simulink model "ofdm_txrx_alamouti"  (Simulink 6.3)
settings: Solver=ode45  StartTime=0.0  StopTime=45000  RelTol=1e-3
InitFcn:
  | ofdm_rx_alamouti_init
StartFcn:
  | ofdm_rx_alamouti_init
values from neighbouring files (symbols this model uses):
  CPLength = 16    [ofdm_tx_alamouti_init.m]
  CRCPolynomial = hex2dec('04c11db7')    [calcTxCRC.m]    (also in ofdm_tx_alamouti_init.m)
  hi = zeros(1,length(cci))    [ofdm_rx_alamouti_init.m]
  hr = zeros(1,length(ccr))    [ofdm_rx_alamouti_init.m]
  max_CP_length = 16    [ofdm_tx_alamouti_init.m]
  max_num_baseRateSymbols = 31    [ofdm_tx_alamouti_init.m]
  max_num_subcarriers = 64    [ofdm_tx_alamouti_init.m]
  max_num_trainingSymbols = 15    [ofdm_tx_alamouti_init.m]
  max_OFDM_symbols = 2047    [ofdm_tx_alamouti_init.m]
  modConstellation_qam16 = 0.75*[1 3 -1 -3]./3    [ofdm_tx_alamouti_init.m]
  modConstellation_qam256 = 0.9375*[3 1 5 7 11 9 13 15 -3 -1 -5 -7 -11 -9 -13 -15]./15    [ofdm_tx_alamouti_init.m]
  modConstellation_qam64 = 0.875*[3 1 5 7 -3 -1 -5 -7]./7    [ofdm_tx_alamouti_init.m]
  modConstellation_qpsk = [1 -1]./sqrt(2)    [ofdm_tx_alamouti_init.m]
  numBaseRateSymbols = 2    [ofdm_tx_alamouti_init.m]
  numFullRateSymbols = numFullRateSymbols + mod(numFullRateSymbols, 2)    [ofdm_tx_alamouti_init.m]
  numSubcarriers = 64    [ofdm_tx_alamouti_init.m]
  numTrainingSymbols = 4    [ofdm_tx_alamouti_init.m]
  pkt_crossCorr_thresh = 0.7    [ofdm_rx_alamouti_init.m]
  pkt_energy_thresh = 0    [ofdm_rx_alamouti_init.m]
  pkt_numPayloadBytes = 24+4+20+20+500    (= 568)    [ofdm_tx_alamouti_init.m]
  pktTiming_count_payloadStart = pktTiming_count_lsStart + pktTiming_ls_num*pktTiming_ls_length    [ofdm_rx_init_packetTimingControl.m]
  preamble = 6*[0 shortsyms_10 longsyms_2]    [ofdm_tx_alamouti_init.m]
  preamble_I = real(preamble)    [ofdm_tx_alamouti_init.m]
  preamble_Q = imag(preamble)    [ofdm_tx_alamouti_init.m]
  RAM_init_size = 4096    [ofdm_tx_alamouti_init.m]
  rx_longCorrCountLoad = 251-16    (= 235)    [ofdm_rx_alamouti_init.m]
  rx_longCorrThresh = 3000    [ofdm_rx_alamouti_init.m]
  RxDataScrambling_Seq = [40 198 78 63 82 173 102 245 48 111 172 115 147 230 216 93 72 65 62 2 205 242 122 90 128 83 105 97 73 10 5 252]    [ofdm_tx_alamouti_init.m]
  simOnly_numSamples = length(preamble)+( (numSubcarriers+CPLength)*(numTrainingSymbols + numBaseRateSymbols + numFullRateSymbols) )    [ofdm_tx_alamouti_init.m]
  train = [train train]    [ofdm_tx_alamouti_init.m]
  TxDataScrambling_Seq = [40 198 78 63 82 173 102 245 48 111 172 115 147 230 216 93 72 65 62 2 205 242 122 90 128 83 105 97 73 10 5 252]    [ofdm_tx_alamouti_init.m]
undefined symbols (not defined in the file or in its folder): CRC_table_gen, gcbh, interpFilter_4, xlBlockLoadCallback
libraries: xbsIndex_r4 x4373, dspmlti4 x4, dspsigops x4, commchan3 x2, simulink x2, commrflib2 x2, commsink2 x2, dspsrcs4 x1
(part 13 of 39)

== system ofdm_txrx_alamouti/OFDM Rx Alamouti/Equalizer & Packetizer/Phase Adjust/ComplexMult_x1 ==
  Re[A] : Inport
  Im[A] : Inport  Port=2
  Re[B] : Inport  Port=3
  Im[B] : Inport  Port=4
  Add1 : xbsIndex_r4/AddSub  mode=Addition  use_carryin=off  use_carryout=off  en=off  latency=0  precision=User Defined  arith_type="Signed  (2's comp)"  n_bits=18  bin_pt=15  quantization=Truncate  overflow=Flag as error  dbl_ovrd=off  use_behavioral_HDL=on  pipelined=off  use_rpm=on  block_type=addsub
      settings: xl_area=[9 0 0 16 0 0 0]  block_version=VER_STRING_GOES_HERE
  Add2 : xbsIndex_r4/AddSub  mode=Addition  use_carryin=off  use_carryout=off  en=off  latency=0  precision=User Defined  arith_type="Signed  (2's comp)"  n_bits=18  bin_pt=15  quantization=Truncate  overflow=Flag as error  dbl_ovrd=off  use_behavioral_HDL=on  pipelined=off  use_rpm=on  block_type=addsub
      settings: xl_area=[9 0 0 16 0 0 0]  block_version=VER_STRING_GOES_HERE
  Counter1 : xbsIndex_r4/Counter  Orientation=down  cnt_type=Free Running  cnt_to=4  operation=Up  start_count=0  cnt_by_val=1  arith_type=Unsigned  n_bits=1  bin_pt=0  load_pin=off  rst=off  en=off  explicit_period=on  period=1  dbl_ovrd=off  use_behavioral_HDL=off  use_rpm=on  block_type=counter
      settings: xl_area=[3 3 0 4 0 0 0]  block_version=VER_STRING_GOES_HERE
  Delay3 : xbsIndex_r4/Delay  en=off  latency=1  dbl_ovrd=off  reg_retiming=off  block_type=delay
      settings: infoedit=Hardware notes: A delay line is a chain, each link of which is an SRL16 followed by a flip-flop. If register retiming is enabled, the delay line is a chain of flip-flops.  xl_area=[8 16 0 0 0 0 0]  block_version=VER_STRING_GOES_HERE
  Delay5 : xbsIndex_r4/Delay  en=off  latency=1  dbl_ovrd=off  reg_retiming=off  block_type=delay
      settings: infoedit=Hardware notes: A delay line is a chain, each link of which is an SRL16 followed by a flip-flop. If register retiming is enabled, the delay line is a chain of flip-flops.  xl_area=[8 16 0 0 0 0 0]  block_version=VER_STRING_GOES_HERE
  Down Sample1 : xbsIndex_r4/Down Sample  sample_ratio=2  sample_phase=First Value of Frame  en=off  latency=1  dbl_ovrd=off  block_type=dsamp
      settings: xl_area=[9 17 0 0 0 0 0]  block_version=VER_STRING_GOES_HERE
  Down Sample3 : xbsIndex_r4/Down Sample  sample_ratio=2  sample_phase=First Value of Frame  en=off  latency=1  dbl_ovrd=off  block_type=dsamp
      settings: xl_area=[9 17 0 0 0 0 0]  block_version=VER_STRING_GOES_HERE
  Mult1 : xbsIndex_r4/Mult  precision=User Defined  arith_type="Signed  (2's comp)"  n_bits=18  bin_pt=16  quantization=Truncate  overflow=Flag as error  en=off  latency=1  dbl_ovrd=off  use_behavioral_HDL=off  use_embedded=on  opt=Speed  optimum_pipeline=off  use_rpm=on  placement_style=Rectangular shape  pipeline=off  block_type=mult
      settings: infoedit=Hardware notes: To use the internal pipeline stage of the dedicated multiplier you must select 'Pipeline for maximum performance'.  xl_area=[14 27 0 27 0 1 0]  block_version=VER_STRING_GOES_HERE
  Mult2 : xbsIndex_r4/Mult  precision=User Defined  arith_type="Signed  (2's comp)"  n_bits=18  bin_pt=16  quantization=Truncate  overflow=Flag as error  en=off  latency=1  dbl_ovrd=off  use_behavioral_HDL=off  use_embedded=on  opt=Speed  optimum_pipeline=off  use_rpm=on  placement_style=Rectangular shape  pipeline=off  block_type=mult
      settings: infoedit=Hardware notes: To use the internal pipeline stage of the dedicated multiplier you must select 'Pipeline for maximum performance'.  xl_area=[14 27 0 27 0 1 0]  block_version=VER_STRING_GOES_HERE
  Mux{2..5} (4 alike) : xbsIndex_r4/Mux  inputs=2  en=off  latency=0  precision=Full  arith_type="Signed  (2's comp)"  n_bits=16  bin_pt=13  quantization=Truncate  overflow=Wrap  dbl_ovrd=off  block_type=mux
      settings: xl_area=[14 0 0 28 0 0 0]  block_version=VER_STRING_GOES_HERE
  Negate2 : xbsIndex_r4/Negate  precision=Full  arith_type="Signed  (2's comp)"  n_bits=14  bin_pt=13  quantization=Truncate  overflow=Flag as error  en=off  latency=0  dbl_ovrd=off  block_type=negate
      settings: xl_area=[7 0 0 14 0 0 0]  block_version=VER_STRING_GOES_HERE
  Up Sample{5..8} (4 alike) : xbsIndex_r4/Up Sample  sample_ratio=2  copy_samples=on  dbl_ovrd=off  block_type=usamp
      settings: infoedit="Up samples input data.  Inserted values  can be zeros or copies of the most recent input sample.<P><P>Hardware notes: No hardware is needed if inserted values are copies of the input sample; otherwise, a mux and single bit flip-flop are used."  xl_area=[0 0 0 0 0 0 0]  block_version=VER_STRING_GOES_HERE
  Re : Outport
  Im : Outport  Port=2
  wires:
  Down Sample1/1 -> Im/1
  Down Sample3/1 -> Re/1
  Add2/1 -> Down Sample3/1
  Add1/1 -> Down Sample1/1
  Delay5/1 -> Add1/2
  Mult2/1 -> Add1/1, Delay5/1
  Negate2/1 -> Mux3/3
  Im[B]/1 -> Up Sample6/1
  Re[B]/1 -> Up Sample8/1
  Im[A]/1 -> Up Sample5/1
  Re[A]/1 -> Up Sample7/1
  Up Sample8/1 -> Mux4/3, Mux2/2
  Up Sample7/1 -> Mux5/2, Mux3/2
  Mux4/1 -> Mult2/2
  Mux5/1 -> Mult2/1
  Mux2/1 -> Mult1/2
  Mux3/1 -> Mult1/1
  Up Sample6/1 -> Mux4/2, Mux2/3
  Up Sample5/1 -> Mux5/3, Negate2/1
  Mult1/1 -> Delay3/1, Add2/1
  Delay3/1 -> Add2/2
  Counter1/1 -> Mux4/1, Mux5/1, Mux2/1, Mux3/1

== system ofdm_txrx_alamouti/OFDM Rx Alamouti/Equalizer & Packetizer/Phase Adjust/ComplexMult_x2 ==
  Re[A] : Inport
  Im[A] : Inport  Port=2
  Re[B] : Inport  Port=3
  Im[B] : Inport  Port=4
  Add1 : xbsIndex_r4/AddSub  mode=Addition  use_carryin=off  use_carryout=off  en=off  latency=0  precision=User Defined  arith_type="Signed  (2's comp)"  n_bits=18  bin_pt=15  quantization=Truncate  overflow=Flag as error  dbl_ovrd=off  use_behavioral_HDL=on  pipelined=off  use_rpm=on  block_type=addsub
      settings: xl_area=[9 0 0 16 0 0 0]  block_version=VER_STRING_GOES_HERE
  Add2 : xbsIndex_r4/AddSub  mode=Addition  use_carryin=off  use_carryout=off  en=off  latency=0  precision=User Defined  arith_type="Signed  (2's comp)"  n_bits=18  bin_pt=15  quantization=Truncate  overflow=Flag as error  dbl_ovrd=off  use_behavioral_HDL=on  pipelined=off  use_rpm=on  block_type=addsub
      settings: xl_area=[9 0 0 16 0 0 0]  block_version=VER_STRING_GOES_HERE
  Counter1 : xbsIndex_r4/Counter  Orientation=down  cnt_type=Free Running  cnt_to=4  operation=Up  start_count=0  cnt_by_val=1  arith_type=Unsigned  n_bits=1  bin_pt=0  load_pin=off  rst=off  en=off  explicit_period=on  period=1  dbl_ovrd=off  use_behavioral_HDL=off  use_rpm=on  block_type=counter
      settings: xl_area=[3 3 0 4 0 0 0]  block_version=VER_STRING_GOES_HERE
  Delay3 : xbsIndex_r4/Delay  en=off  latency=1  dbl_ovrd=off  reg_retiming=off  block_type=delay
      settings: infoedit=Hardware notes: A delay line is a chain, each link of which is an SRL16 followed by a flip-flop. If register retiming is enabled, the delay line is a chain of flip-flops.  xl_area=[8 16 0 0 0 0 0]  block_version=VER_STRING_GOES_HERE
  Delay5 : xbsIndex_r4/Delay  en=off  latency=1  dbl_ovrd=off  reg_retiming=off  block_type=delay
      settings: infoedit=Hardware notes: A delay line is a chain, each link of which is an SRL16 followed by a flip-flop. If register retiming is enabled, the delay line is a chain of flip-flops.  xl_area=[8 16 0 0 0 0 0]  block_version=VER_STRING_GOES_HERE
  Down Sample1 : xbsIndex_r4/Down Sample  sample_ratio=2  sample_phase=First Value of Frame  en=off  latency=1  dbl_ovrd=off  block_type=dsamp
      settings: xl_area=[9 17 0 0 0 0 0]  block_version=VER_STRING_GOES_HERE
  Down Sample3 : xbsIndex_r4/Down Sample  sample_ratio=2  sample_phase=First Value of Frame  en=off  latency=1  dbl_ovrd=off  block_type=dsamp
      settings: xl_area=[9 17 0 0 0 0 0]  block_version=VER_STRING_GOES_HERE
  Mult1 : xbsIndex_r4/Mult  precision=User Defined  arith_type="Signed  (2's comp)"  n_bits=18  bin_pt=16  quantization=Truncate  overflow=Flag as error  en=off  latency=1  dbl_ovrd=off  use_behavioral_HDL=off  use_embedded=on  opt=Speed  optimum_pipeline=off  use_rpm=on  placement_style=Rectangular shape  pipeline=off  block_type=mult
      settings: infoedit=Hardware notes: To use the internal pipeline stage of the dedicated multiplier you must select 'Pipeline for maximum performance'.  xl_area=[14 27 0 27 0 1 0]  block_version=VER_STRING_GOES_HERE
  Mult2 : xbsIndex_r4/Mult  precision=User Defined  arith_type="Signed  (2's comp)"  n_bits=18  bin_pt=16  quantization=Truncate  overflow=Flag as error  en=off  latency=1  dbl_ovrd=off  use_behavioral_HDL=off  use_embedded=on  opt=Speed  optimum_pipeline=off  use_rpm=on  placement_style=Rectangular shape  pipeline=off  block_type=mult
      settings: infoedit=Hardware notes: To use the internal pipeline stage of the dedicated multiplier you must select 'Pipeline for maximum performance'.  xl_area=[14 27 0 27 0 1 0]  block_version=VER_STRING_GOES_HERE
  Mux{2..5} (4 alike) : xbsIndex_r4/Mux  inputs=2  en=off  latency=0  precision=Full  arith_type="Signed  (2's comp)"  n_bits=16  bin_pt=13  quantization=Truncate  overflow=Wrap  dbl_ovrd=off  block_type=mux
      settings: xl_area=[14 0 0 28 0 0 0]  block_version=VER_STRING_GOES_HERE
  Negate2 : xbsIndex_r4/Negate  precision=Full  arith_type="Signed  (2's comp)"  n_bits=14  bin_pt=13  quantization=Truncate  overflow=Flag as error  en=off  latency=0  dbl_ovrd=off  block_type=negate
      settings: xl_area=[7 0 0 14 0 0 0]  block_version=VER_STRING_GOES_HERE
  Up Sample{5..8} (4 alike) : xbsIndex_r4/Up Sample  sample_ratio=2  copy_samples=on  dbl_ovrd=off  block_type=usamp
      settings: infoedit="Up samples input data.  Inserted values  can be zeros or copies of the most recent input sample.<P><P>Hardware notes: No hardware is needed if inserted values are copies of the input sample; otherwise, a mux and single bit flip-flop are used."  xl_area=[0 0 0 0 0 0 0]  block_version=VER_STRING_GOES_HERE
  Re : Outport
  Im : Outport  Port=2
  wires:
  Counter1/1 -> Mux3/1, Mux2/1, Mux5/1, Mux4/1
  Delay3/1 -> Add2/2
  Mult1/1 -> Add2/1, Delay3/1
  Up Sample5/1 -> Negate2/1, Mux5/3
  Up Sample6/1 -> Mux2/3, Mux4/2
  Mux3/1 -> Mult1/1
  Mux2/1 -> Mult1/2
  Mux5/1 -> Mult2/1
  Mux4/1 -> Mult2/2
  Up Sample7/1 -> Mux3/2, Mux5/2
  Up Sample8/1 -> Mux2/2, Mux4/3
  Re[A]/1 -> Up Sample7/1
  Im[A]/1 -> Up Sample5/1
  Re[B]/1 -> Up Sample8/1
  Im[B]/1 -> Up Sample6/1
  Negate2/1 -> Mux3/3
  Mult2/1 -> Delay5/1, Add1/1
  Delay5/1 -> Add1/2
  Add1/1 -> Down Sample1/1
  Add2/1 -> Down Sample3/1
  Down Sample3/1 -> Re/1
  Down Sample1/1 -> Im/1

== system ofdm_txrx_alamouti/OFDM Rx Alamouti/Equalizer & Packetizer/Phase Adjust/Phase Calc ==
  Phase : Inport
  Convert4 : xbsIndex_r4/Convert  arith_type="Signed  (2's comp)"  n_bits=15  bin_pt=10  quantization=Truncate  overflow=Flag as error  latency=0  dbl_ovrd=off  pipeline=off  block_type=convert
      settings: xl_area=[0, 0, 0, 0, 0, 0, 0]  block_version=VER_STRING_GOES_HERE
  Down Sample1 : xbsIndex_r4/Down Sample  sample_ratio=2  sample_phase="Last Value of Frame  (most efficient)"  en=off  latency=1  dbl_ovrd=off  block_type=dsamp
      settings: block_version=VER_STRING_GOES_HERE
  Mult1 : xbsIndex_r4/Mult  precision=User Defined  arith_type="Signed  (2's comp)"  n_bits=12  bin_pt=10  quantization=Truncate  overflow=Flag as error  en=off  latency=1  dbl_ovrd=off  use_behavioral_HDL=off  use_embedded=on  opt=Speed  optimum_pipeline=off  use_rpm=off  placement_style=Rectangular shape  pipeline=on  block_type=mult
      settings: infoedit=Hardware notes: To use the internal pipeline stage of the dedicated multiplier you must select 'Pipeline for maximum performance'.  block_version=VER_STRING_GOES_HERE
  Rx_PhaseNoiseTrack_K : xbsIndex_r4/From Register  shared_memory_name='Rx_PhaseNoiseTrack_K'  init=Rx_PhaseNoiseTrack_K  period=1  ownership=Locally owned and initialized  arith_type=Unsigned  n_bits=32  bin_pt=26  dbl_ovrd=off  block_type=fromreg
      settings: AttributesFormatString=<< %<shared_memory_name> >>  infoedit="Register block that reads data to a shared memory register.  Delay of one sample period."  block_version=VER_STRING_GOES_HERE
  " Phase" : Outport
  wires:
  Phase/1 -> Convert4/1
  Down Sample1/1 -> Mult1/2
  Rx_PhaseNoiseTrack_K/1 -> Down Sample1/1
  Mult1/1 -> " Phase"/1
  Convert4/1 -> Mult1/1

== system ofdm_txrx_alamouti/OFDM Rx Alamouti/Equalizer & Packetizer/Phase Noise Tracker/Coarse Tracking ==
  Phase Err : Inport
  Rst : Inport  Port=2
  Vin : Inport  Port=3
  Accumulator1 : xbsIndex_r4/Accumulator  operation=Add  n_bits=32  overflow=Wrap  scale=1  rst=on  hasbypass=off  en=on  dbl_ovrd=off  use_behavioral_HDL=on  block_type=accum
      settings: infoedit="Adder or subtractor-based accumulator.   Output type and binary point position match the input.<P><P>Hardware notes: When \"Reinitialize with input 'b' on reset\" is selected, the accumulator is forced to run at the system rate even if the input 'b' is running at a slower rate."  block_version=VER_STRING_GOES_HERE
  AddSub1 : xbsIndex_r4/AddSub  mode=Subtraction  use_carryin=off  use_carryout=off  en=off  latency=0  precision=User Defined  arith_type="Signed  (2's comp)"  n_bits=32  bin_pt=28  quantization=Truncate  overflow=Wrap  dbl_ovrd=off  use_behavioral_HDL=off  pipelined=off  use_rpm=on  block_type=addsub
      settings: block_version=VER_STRING_GOES_HERE
  Coarse Phase Tracker : Scope  NumInputPorts=6
      settings: YMin=-1~-1~-1~-1~-1~-1  YMax=1~1~1~1~1~1  SaveName=ScopeData40
  Delay : xbsIndex_r4/Delay  en=off  latency=3  dbl_ovrd=off  reg_retiming=off  block_type=delay
      settings: infoedit=Hardware notes: A delay line is a chain, each link of which is an SRL16 followed by a flip-flop. If register retiming is enabled, the delay line is a chain of flip-flops.  block_version=VER_STRING_GOES_HERE
  Delay1 : xbsIndex_r4/Delay  en=off  latency=1  dbl_ovrd=off  reg_retiming=off  block_type=delay
      settings: infoedit=Hardware notes: A delay line is a chain, each link of which is an SRL16 followed by a flip-flop. If register retiming is enabled, the delay line is a chain of flip-flops.  block_version=VER_STRING_GOES_HERE
  Display : Display
  Down Sample2 : xbsIndex_r4/Down Sample  sample_ratio=2  sample_phase="Last Value of Frame  (most efficient)"  en=off  latency=1  dbl_ovrd=off  block_type=dsamp
      settings: block_version=VER_STRING_GOES_HERE
  Gateway Out{1..6} (6 alike) : xbsIndex_r4/Gateway Out  hdl_port=off  timing_constraint=None  locs_specified=off  LOCs={}  block_type=gatewayout
      settings: infoedit="Gateway out block.  Converts Xilinx fixed point inputs into ouputs of type Simulink integer, double, or fixed point.<P><P>Hardware notes:  In hardware these blocks become top level output ports or are discarded, depending on how they are configured."  block_version=VER_STRING_GOES_HERE
  Mult2 : xbsIndex_r4/Mult  precision=User Defined  arith_type="Signed  (2's comp)"  n_bits=32  bin_pt=28  quantization=Truncate  overflow=Wrap  en=off  latency=3  dbl_ovrd=off  use_behavioral_HDL=off  use_embedded=on  opt=Speed  optimum_pipeline=off  use_rpm=off  placement_style=Rectangular shape  pipeline=on  block_type=mult
      settings: infoedit=Hardware notes: To use the internal pipeline stage of the dedicated multiplier you must select 'Pipeline for maximum performance'.  block_version=VER_STRING_GOES_HERE
  Register1 : xbsIndex_r4/Register  Orientation=left  init=0  rst=on  en=on  dbl_ovrd=off  block_type=register
      settings: block_version=8.2.02
  Register2 : xbsIndex_r4/Register  init=0  rst=on  en=on  dbl_ovrd=off  block_type=register
      settings: block_version=8.2.02
  Rx_PhaseNoiseTrack_Kalpha : xbsIndex_r4/From Register  shared_memory_name='Rx_PhaseNoiseTrack_Kalpha'  init=Rx_PhaseNoiseTrack_Kalpha  period=1  ownership=Locally owned and initialized  arith_type=Unsigned  n_bits=32  bin_pt=32  dbl_ovrd=off  block_type=fromreg
      settings: AttributesFormatString=<< %<shared_memory_name> >>  infoedit="Register block that reads data to a shared memory register.  Delay of one sample period."  block_version=VER_STRING_GOES_HERE
  phi : Outport
  theta_coarse : Outport  Port=2
  vout : Outport  Port=3
  wires:
  Gateway Out6/1 -> Coarse Phase Tracker/6
  Gateway Out5/1 -> Coarse Phase Tracker/5
  Gateway Out4/1 -> Coarse Phase Tracker/4
  Gateway Out3/1 -> Coarse Phase Tracker/3
  Gateway Out1/1 -> Coarse Phase Tracker/2
  Gateway Out2/1 -> Coarse Phase Tracker/1
  Phase Err/1 -> AddSub1/1, Gateway Out2/1
  Rx_PhaseNoiseTrack_Kalpha/1 -> Down Sample2/1, Display/1
  Down Sample2/1 -> Mult2/2
  Mult2/1 -> Accumulator1/1
  Delay/1 -> Accumulator1/3
  Accumulator1/1 -> theta_coarse/1, Register1/1, Gateway Out3/1
  AddSub1/1 -> Register2/1
  Register2/1 -> phi/1, Mult2/1, Gateway Out1/1
  Rst/1 -> Register2/2, Register1/2, Accumulator1/2, Gateway Out6/1
  Vin/1 -> Register2/3, Register1/3, Delay/1, Delay1/1, Gateway Out5/1
  Register1/1 -> AddSub1/2, Gateway Out4/1
  Delay1/1 -> vout/1

== system ofdm_txrx_alamouti/OFDM Rx Alamouti/Equalizer & Packetizer/Phase Noise Tracker/Fine Tracking ==
  note: Overflow potential before/after accumulator?
  phi : Inport
  theta_coarse : Inport  Port=2
  Vin : Inport  Port=3
  Rst : Inport  Port=4
  Sym Index : Inport  Port=5
  Accumulator1 : xbsIndex_r4/Accumulator  operation=Add  n_bits=32  overflow=Flag as error  scale=1  rst=on  hasbypass=off  en=on  dbl_ovrd=off  use_behavioral_HDL=on  block_type=accum
      settings: infoedit="Adder or subtractor-based accumulator.   Output type and binary point position match the input.<P><P>Hardware notes: When \"Reinitialize with input 'b' on reset\" is selected, the accumulator is forced to run at the system rate even if the input 'b' is running at a slower rate."  block_version=VER_STRING_GOES_HERE
  AddSub1 : xbsIndex_r4/AddSub  mode=Addition  use_carryin=off  use_carryout=off  en=off  latency=0  precision=User Defined  arith_type="Signed  (2's comp)"  n_bits=32  bin_pt=28  quantization=Truncate  overflow=Wrap  dbl_ovrd=off  use_behavioral_HDL=off  pipelined=off  use_rpm=on  block_type=addsub
      settings: block_version=VER_STRING_GOES_HERE
  AddSub2 : xbsIndex_r4/AddSub  mode=Addition  use_carryin=off  use_carryout=off  en=off  latency=0  precision=User Defined  arith_type="Signed  (2's comp)"  n_bits=32  bin_pt=28  quantization=Truncate  overflow=Wrap  dbl_ovrd=off  use_behavioral_HDL=off  pipelined=off  use_rpm=on  block_type=addsub
      settings: block_version=VER_STRING_GOES_HERE
  Constant7 : xbsIndex_r4/Constant  arith_type=Unsigned  const=0.5  n_bits=4  bin_pt=2  explicit_period=on  period=1  equ=P=C  opselect=C  inp2=PCIN>>17  opr=+  inp1=P  carry=CIN  dbl_ovrd=off  block_type=constant
      settings: block_version=VER_STRING_GOES_HERE
  Delay : xbsIndex_r4/Delay  en=off  latency=3  dbl_ovrd=off  reg_retiming=off  block_type=delay
      settings: infoedit=Hardware notes: A delay line is a chain, each link of which is an SRL16 followed by a flip-flop. If register retiming is enabled, the delay line is a chain of flip-flops.  block_version=VER_STRING_GOES_HERE
  Delay1 : xbsIndex_r4/Delay  en=off  latency=4  dbl_ovrd=off  reg_retiming=off  block_type=delay
      settings: infoedit=Hardware notes: A delay line is a chain, each link of which is an SRL16 followed by a flip-flop. If register retiming is enabled, the delay line is a chain of flip-flops.  block_version=VER_STRING_GOES_HERE
  Display : Display
  Display1 : Display
  Down Sample1 : xbsIndex_r4/Down Sample  sample_ratio=2  sample_phase="Last Value of Frame  (most efficient)"  en=off  latency=1  dbl_ovrd=off  block_type=dsamp
      settings: block_version=VER_STRING_GOES_HERE
  Down Sample2 : xbsIndex_r4/Down Sample  sample_ratio=2  sample_phase="Last Value of Frame  (most efficient)"  en=off  latency=1  dbl_ovrd=off  block_type=dsamp
      settings: block_version=VER_STRING_GOES_HERE
  Fine Phase Tracker : Scope  NumInputPorts=6
      settings: YMin=-1~-1~-1~-1~-1~-1  YMax=1~1~1~1~1~1  SaveName=ScopeData40
  Gateway Out{1..6} (6 alike) : xbsIndex_r4/Gateway Out  hdl_port=off  timing_constraint=None  locs_specified=off  LOCs={}  block_type=gatewayout
      settings: infoedit="Gateway out block.  Converts Xilinx fixed point inputs into ouputs of type Simulink integer, double, or fixed point.<P><P>Hardware notes:  In hardware these blocks become top level output ports or are discarded, depending on how they are configured."  block_version=VER_STRING_GOES_HERE
  Mult1 : xbsIndex_r4/Mult  precision=User Defined  arith_type="Signed  (2's comp)"  n_bits=32  bin_pt=28  quantization=Truncate  overflow=Wrap  en=off  latency=3  dbl_ovrd=off  use_behavioral_HDL=off  use_embedded=on  opt=Speed  optimum_pipeline=off  use_rpm=off  placement_style=Rectangular shape  pipeline=on  block_type=mult
      settings: infoedit=Hardware notes: To use the internal pipeline stage of the dedicated multiplier you must select 'Pipeline for maximum performance'.  block_version=VER_STRING_GOES_HERE
  Mult2 : xbsIndex_r4/Mult  precision=User Defined  arith_type="Signed  (2's comp)"  n_bits=32  bin_pt=24  quantization=Truncate  overflow=Wrap  en=off  latency=3  dbl_ovrd=off  use_behavioral_HDL=off  use_embedded=on  opt=Speed  optimum_pipeline=off  use_rpm=off  placement_style=Rectangular shape  pipeline=on  block_type=mult
      settings: infoedit=Hardware notes: To use the internal pipeline stage of the dedicated multiplier you must select 'Pipeline for maximum performance'.  block_version=VER_STRING_GOES_HERE
  Mult3 : xbsIndex_r4/Mult  precision=User Defined  arith_type="Signed  (2's comp)"  n_bits=32  bin_pt=28  quantization=Truncate  overflow=Wrap  en=off  latency=3  dbl_ovrd=off  use_behavioral_HDL=off  use_embedded=on  opt=Speed  optimum_pipeline=off  use_rpm=off  placement_style=Rectangular shape  pipeline=on  block_type=mult
      settings: infoedit=Hardware notes: To use the internal pipeline stage of the dedicated multiplier you must select 'Pipeline for maximum performance'.  block_version=VER_STRING_GOES_HERE
  ROM : xbsIndex_r4/ROM  depth=floor(max_OFDM_symbols/2)  initVector=1./[1:floor(max_OFDM_symbols/2)]  distributed_mem=Distributed memory  rst=off  init_reg=0  en=off  latency=1  arith_type=Unsigned  n_bits=8  bin_pt=8  dbl_ovrd=off  optimize=Area  use_rpm=on  block_type=sprom
      settings: block_version=8.2.02
  Register2 : xbsIndex_r4/Register  init=0  rst=on  en=on  dbl_ovrd=off  block_type=register
      settings: block_version=8.2.02
  Slice9 : xbsIndex_r4/Slice  nbits=ceil(log2(max_OFDM_symbols))-1  boolean_output=off  mode=Upper Bit Location + Width  bit1=0  base1=MSB of Input  bit0=0  base0=LSB of Input  dbl_ovrd=off  block_type=slice
      settings: infoedit="Extracts a given range of bits from each input sample and presents it at the output.  The output type is ordinarily unsigned with binary point at zero, but can be Boolean when the slice is one bit wide.<P><P>Hardware notes: In hardware this block costs nothing."  block_version=VER_STRING_GOES_HERE
  theta : Outport
  wires:
  AddSub1/1 -> Register2/1
  Delay/1 -> Accumulator1/3, Delay1/1
  Mult2/1 -> Accumulator1/1
  Down Sample2/1 -> Mult2/2
  theta_coarse/1 -> AddSub1/1, Gateway Out1/1
  Gateway Out2/1 -> Fine Phase Tracker/1
  Gateway Out1/1 -> Fine Phase Tracker/2
  Gateway Out3/1 -> Fine Phase Tracker/3
  Gateway Out4/1 -> Fine Phase Tracker/4
  Gateway Out5/1 -> Fine Phase Tracker/5
  Gateway Out6/1 -> Fine Phase Tracker/6
  phi/1 -> Mult2/1, Mult1/2, Gateway Out2/1
  Down Sample1/1 -> Mult1/1
  Constant7/1 -> Down Sample1/1, Display1/1, Display/1, Down Sample2/1
  Mult1/1 -> AddSub2/1
  Accumulator1/1 -> Mult3/1
  ROM/1 -> Mult3/2, Gateway Out5/1
  Sym Index/1 -> Slice9/1
  Mult3/1 -> AddSub2/2, Gateway Out6/1
  AddSub2/1 -> AddSub1/2, Gateway Out3/1
  Rst/1 -> Accumulator1/2, Register2/2
  Vin/1 -> Delay/1
  Delay1/1 -> Register2/3
  Register2/1 -> Gateway Out4/1, theta/1
  Slice9/1 -> ROM/1

== system ofdm_txrx_alamouti/OFDM Rx Alamouti/Equalizer & Packetizer/Pilot_Extractor/Phase Calc & Combining ==
  Rst : Inport
  I : Inport  Port=2
  Q : Inport  Port=3
  mag(H) : Inport  Port=4
  Vin : Inport  Port=5
  1/sum(mag(H)) : Subsystem
  Accumulator : xbsIndex_r4/Accumulator  operation=Add  n_bits=24  overflow=Flag as error  scale=1  rst=on  hasbypass=off  en=on  dbl_ovrd=off  use_behavioral_HDL=on  block_type=accum
      settings: infoedit="Adder or subtractor-based accumulator.   Output type and binary point position match the input.<P><P>Hardware notes: When \"Reinitialize with input 'b' on reset\" is selected, the accumulator is forced to run at the system rate even if the input 'b' is running at a slower rate."  block_version=8.2.02
  Constant : xbsIndex_r4/Constant  arith_type=Unsigned  const=0  n_bits=1  bin_pt=0  explicit_period=off  period=1  equ=P=C  opselect=C  inp2=PCIN>>17  opr=+  inp1=P  carry=CIN  dbl_ovrd=off  block_type=constant
      settings: block_version=8.2.02
  Counter : xbsIndex_r4/Counter  cnt_type=Free Running  cnt_to=Inf  operation=Up  start_count=0  cnt_by_val=1  arith_type=Unsigned  n_bits=3  bin_pt=0  load_pin=off  rst=on  en=on  explicit_period=off  period=1  dbl_ovrd=off  use_behavioral_HDL=off  use_rpm=off  block_type=counter
      settings: block_version=8.2.02
  Delay1 : xbsIndex_r4/Delay  en=off  latency=8  dbl_ovrd=off  reg_retiming=off  block_type=delay
      settings: infoedit=Hardware notes: A delay line is a chain, each link of which is an SRL16 followed by a flip-flop. If register retiming is enabled, the delay line is a chain of flip-flops.  block_version=8.2.02
  Delay2 : xbsIndex_r4/Delay  en=off  latency=2  dbl_ovrd=off  reg_retiming=off  block_type=delay
      settings: infoedit=Hardware notes: A delay line is a chain, each link of which is an SRL16 followed by a flip-flop. If register retiming is enabled, the delay line is a chain of flip-flops.  block_version=8.2.02
  Delay3 : xbsIndex_r4/Delay  en=off  latency=8  dbl_ovrd=off  reg_retiming=off  block_type=delay
      settings: infoedit=Hardware notes: A delay line is a chain, each link of which is an SRL16 followed by a flip-flop. If register retiming is enabled, the delay line is a chain of flip-flops.  block_version=8.2.02
  Delay5 : xbsIndex_r4/Delay  en=off  latency=3  dbl_ovrd=off  reg_retiming=off  block_type=delay
      settings: infoedit=Hardware notes: A delay line is a chain, each link of which is an SRL16 followed by a flip-flop. If register retiming is enabled, the delay line is a chain of flip-flops.  block_version=8.2.02
  Gateway Out{1..3,7..11} (8 alike) : xbsIndex_r4/Gateway Out  hdl_port=off  timing_constraint=None  locs_specified=off  LOCs={}  block_type=gatewayout
      settings: infoedit="Gateway out block.  Converts Xilinx fixed point inputs into ouputs of type Simulink integer, double, or fixed point.<P><P>Hardware notes:  In hardware these blocks become top level output ports or are discarded, depending on how they are configured."  block_version=VER_STRING_GOES_HERE
  Logical : xbsIndex_r4/Logical  logical_function=AND  inputs=2  en=off  latency=0  precision=Full  arith_type=Unsigned  n_bits=16  bin_pt=0  align_bp=on  dbl_ovrd=off  block_type=logical
      settings: block_version=8.2.02
  Mult : xbsIndex_r4/Mult  precision=User Defined  arith_type="Signed  (2's comp)"  n_bits=21  bin_pt=16  quantization=Truncate  overflow=Flag as error  en=off  latency=2  dbl_ovrd=off  use_behavioral_HDL=off  use_embedded=on  opt=Speed  optimum_pipeline=off  use_rpm=on  placement_style=Rectangular shape  pipeline=on  block_type=mult
      settings: infoedit=Hardware notes: To use the internal pipeline stage of the dedicated multiplier you must select 'Pipeline for maximum performance'.  block_version=8.2.02
  Mult1 : xbsIndex_r4/Mult  precision=User Defined  arith_type="Signed  (2's comp)"  n_bits=21  bin_pt=16  quantization=Truncate  overflow=Saturate  en=off  latency=2  dbl_ovrd=off  use_behavioral_HDL=off  use_embedded=on  opt=Speed  optimum_pipeline=off  use_rpm=on  placement_style=Rectangular shape  pipeline=on  block_type=mult
      settings: infoedit=Hardware notes: To use the internal pipeline stage of the dedicated multiplier you must select 'Pipeline for maximum performance'.  block_version=8.2.02
  Phase Calc : Subsystem
  Pilot Phase Combining : Scope  NumInputPorts=8
      settings: TimeRange=5000  YMin=-1~-1~-1~2.29892~-1~-0.0190659~-1~-0.0461426  YMax=1~1~1~2.54092~1~-0.0172501~1~-0.041748  SaveName=ScopeData59
  Register : xbsIndex_r4/Register  init=0  rst=on  en=on  dbl_ovrd=off  block_type=register
      settings: block_version=8.2.02
  Relational3 : xbsIndex_r4/Relational  mode=a=b  en=off  latency=0  dbl_ovrd=off  block_type=relational
      settings: xl_area=[6 0 0 11 0 0 0]  block_version=VER_STRING_GOES_HERE
  PhaseEst : Outport
  Vout : Outport  Port=2
  wires:
  I/1 -> Phase Calc/1(I)
  Q/1 -> Phase Calc/2(Q)
  Phase Calc/1(Phase) -> Mult/1
  mag(H)/1 -> Delay3/1, 1/sum(mag(H))/1(mag(H))
  Mult/1 -> Accumulator/1
  Vin/1 -> Delay1/1, 1/sum(mag(H))/2(Vin), Logical/2, Counter/2, Gateway Out1/1
  Delay1/1 -> Delay2/1
  Delay2/1 -> Accumulator/3
  Accumulator/1 -> Mult1/2, Gateway Out9/1
  Gateway Out11/1 -> Pilot Phase Combining/4
  Gateway Out10/1 -> Pilot Phase Combining/5
  Gateway Out9/1 -> Pilot Phase Combining/6
  Gateway Out8/1 -> Pilot Phase Combining/7
  Gateway Out7/1 -> Pilot Phase Combining/8
  Delay3/1 -> Mult/2
  Counter/1 -> Relational3/2, 1/sum(mag(H))/3(PilotNum), Gateway Out3/1
  Rst/1 -> Counter/1, Gateway Out2/1
  Mult1/1 -> PhaseEst/1, Gateway Out7/1
  Register/1 -> Mult1/1
  1/sum(mag(H))/1(Q) -> Register/1, Gateway Out11/1
  1/sum(mag(H))/2(Vout) -> Register/3, Gateway Out10/1, Delay5/1
  Delay5/1 -> Vout/1, Gateway Out8/1
  Constant/1 -> Relational3/1
  Relational3/1 -> Logical/1
  Logical/1 -> Register/2, Accumulator/2
  Gateway Out2/1 -> Pilot Phase Combining/1
  Gateway Out1/1 -> Pilot Phase Combining/2
  Gateway Out3/1 -> Pilot Phase Combining/3

== system ofdm_txrx_alamouti/OFDM Rx Alamouti/Equalizer & Packetizer/Pilot_Extractor/Pilot Descrambling ==
  Sym Index : Inport
  Rst : Inport  Port=2
  "I " : Inport  Port=3
  "Q " : Inport  Port=4
  FFT Index : Inport  Port=5
  Vin : Inport  Port=6
  Gateway Out{7..14} (8 alike) : xbsIndex_r4/Gateway Out  hdl_port=off  timing_constraint=None  locs_specified=off  LOCs={}  block_type=gatewayout
      settings: infoedit="Gateway out block.  Converts Xilinx fixed point inputs into ouputs of type Simulink integer, double, or fixed point.<P><P>Hardware notes:  In hardware these blocks become top level output ports or are discarded, depending on how they are configured."  block_version=VER_STRING_GOES_HERE
  Individual Pilots : Scope  NumInputPorts=8
      settings: TimeRange=5000  YMin=-0.25~-0.075~-0.25~-0.075~-0.25~-0.075~-0.25~-0.075  YMax=0~0~0~0~0~0~0~0  SaveName=ScopeData45
  Logical1 : xbsIndex_r4/Logical  logical_function=AND  inputs=2  en=off  latency=0  precision=Full  arith_type=Unsigned  n_bits=8  bin_pt=2  align_bp=on  dbl_ovrd=off  block_type=logical
      settings: xl_area=[2 0 0 2 0 0 0]  block_version=VER_STRING_GOES_HERE
  Pilot_Descrambler : Subsystem
  PilotsEN : Subsystem
  Register{2..9} (8 alike) : xbsIndex_r4/Register  init=0  rst=off  en=on  dbl_ovrd=off  block_type=register
      settings: block_version=VER_STRING_GOES_HERE
  I : Outport
  Q : Outport  Port=2
  PilotEn : Outport  Port=3
  wires:
  Pilot_Descrambler/2(Q) -> Register3/1, Register5/1, Register7/1, Register9/1, Q/1
  Pilot_Descrambler/1(I) -> Register2/1, Register4/1, Register6/1, Register8/1, I/1
  PilotsEN/5(4_EN) -> Register9/2, Register8/2
  PilotsEN/3(2_EN) -> Register5/2, Register4/2
  PilotsEN/2(1_EN) -> Register2/2, Register3/2
  Register9/1 -> Gateway Out13/1
  Register8/1 -> Gateway Out14/1
  Register7/1 -> Gateway Out12/1
  Register6/1 -> Gateway Out7/1
  Register5/1 -> Gateway Out8/1
  Register4/1 -> Gateway Out9/1
  Register3/1 -> Gateway Out10/1
  Register2/1 -> Gateway Out11/1
  Gateway Out13/1 -> Individual Pilots/8
  Gateway Out14/1 -> Individual Pilots/7
  Gateway Out12/1 -> Individual Pilots/6
  Gateway Out7/1 -> Individual Pilots/5
  Gateway Out8/1 -> Individual Pilots/4
  Gateway Out9/1 -> Individual Pilots/3
  Gateway Out10/1 -> Individual Pilots/2
  Gateway Out11/1 -> Individual Pilots/1
  PilotsEN/4(3_EN) -> Register7/2, Register6/2, Pilot_Descrambler/6(Neg Pilot)
  Sym Index/1 -> Pilot_Descrambler/1(Sym Index)
  Rst/1 -> Pilot_Descrambler/2(rst)
  "I "/1 -> Pilot_Descrambler/3("I ")
  "Q "/1 -> Pilot_Descrambler/4("Q ")
  PilotsEN/1(Any_EN) -> Logical1/1
  FFT Index/1 -> PilotsEN/1(xk_index), Pilot_Descrambler/5(FFT Index)
  Logical1/1 -> PilotEn/1
  Vin/1 -> Logical1/2

== system ofdm_txrx_alamouti/OFDM Rx Alamouti/Equalizer & Packetizer/Pilot_Extractor/Pilot Phase Equalizer ==
  SlotSel : Inport
  Y_I : Inport  Port=2
  Y_Q : Inport  Port=3
  H_I : Inport  Port=4
  H_Q : Inport  Port=5
  Bus Selector : Subsystem
  Bus Selector2 : Subsystem
  ComplexMag : Subsystem
  Delay2 : xbsIndex_r4/Delay  en=off  latency=2  dbl_ovrd=off  reg_retiming=off  block_type=delay
      settings: infoedit=Hardware notes: A delay line is a chain, each link of which is an SRL16 followed by a flip-flop. If register retiming is enabled, the delay line is a chain of flip-flops.  xl_area=[0, 0, 0, 0, 0, 0, 0]  block_version=VER_STRING_GOES_HERE
  Gateway Out{1..6} (6 alike) : xbsIndex_r4/Gateway Out  hdl_port=off  timing_constraint=None  locs_specified=off  LOCs={}  block_type=gatewayout
      settings: infoedit="Gateway out block.  Converts Xilinx fixed point inputs into ouputs of type Simulink integer, double, or fixed point.<P><P>Hardware notes:  In hardware these blocks become top level output ports or are discarded, depending on how they are configured."  block_version=VER_STRING_GOES_HERE
  Inverter : xbsIndex_r4/Inverter  en=off  latency=0  dbl_ovrd=off  block_type=inv
      settings: block_version=VER_STRING_GOES_HERE
  Mux{1..4} (4 alike) : xbsIndex_r4/Mux  inputs=2  en=off  latency=0  precision=Full  arith_type="Signed  (2's comp)"  n_bits=16  bin_pt=13  quantization=Truncate  overflow=Wrap  dbl_ovrd=off  block_type=mux
      settings: xl_area=[14 0 0 28 0 0 0]  block_version=VER_STRING_GOES_HERE
  Pilot Extractor : Scope  NumInputPorts=6
      settings: TimeRange=12000  YMin=-0.3~-0.3~-1~-0.3~-1~-0.3  YMax=0.3~0.3~1~0.3~1~0.3  SaveName=ScopeData50
  R0*[H0*] : Subsystem
  R1 *[H1*] : Subsystem
  I : Outport
  Q : Outport  Port=2
  mag(H ) : Outport  Port=3
  wires:
  Bus Selector/4(BA_Q) -> R1 *[H1*]/4(Im[h1]), Mux4/3
  Bus Selector/3(BA_I) -> R1 *[H1*]/3(Re[h1]), Mux2/3
  Bus Selector/2(AA_Q) -> R0*[H0*]/4(Im[h1]), Mux4/2
  Bus Selector/1(AA_I) -> R0*[H0*]/3(Re[h1]), Mux2/2
  Bus Selector2/4(R1_Q) -> R1 *[H1*]/2(Im[y])
  Bus Selector2/3(R1_I) -> R1 *[H1*]/1(Re[y])
  Bus Selector2/2(R0_Q) -> R0*[H0*]/2(Im[y])
  Bus Selector2/1(R0_I) -> R0*[H0*]/1(Re[y])
  R1 *[H1*]/2(Im) -> Mux1/3, Gateway Out6/1
  Y_I/1 -> Bus Selector2/1(Y_I)
  Y_Q/1 -> Bus Selector2/2(Y_Q)
  H_I/1 -> Bus Selector/1(H-I)
  H_Q/1 -> Bus Selector/2(H-Q)
  R0*[H0*]/1(Re) -> Mux3/2, Gateway Out3/1
  R1 *[H1*]/1(Re) -> Mux3/3, Gateway Out2/1
  R0*[H0*]/2(Im) -> Mux1/2, Gateway Out1/1
  SlotSel/1 -> Delay2/1, Mux2/1, Mux4/1, Inverter/1
  Mux3/1 -> I/1, Gateway Out5/1
  Mux1/1 -> Q/1, Gateway Out4/1
  Delay2/1 -> Mux3/1, Mux1/1
  Gateway Out5/1 -> Pilot Extractor/1
  Gateway Out4/1 -> Pilot Extractor/2
  Gateway Out3/1 -> Pilot Extractor/3
  Gateway Out2/1 -> Pilot Extractor/4
  Gateway Out1/1 -> Pilot Extractor/5
  Gateway Out6/1 -> Pilot Extractor/6
  ComplexMag/1(Re) -> mag(H )/1
  Mux2/1 -> ComplexMag/1(Re[A])
  Mux4/1 -> ComplexMag/2(Im[A])

== system ofdm_txrx_alamouti/OFDM Rx Alamouti/Equalizer & Packetizer/Pilot_Extractor/S-R Latch ==
  S : Inport
  R : Inport  Port=2
  Constant2 : xbsIndex_r4/Constant  arith_type=Boolean  const=1  n_bits=1  bin_pt=0  explicit_period=off  period=1  equ=P=C  opselect=C  inp2=PCIN>>17  opr=+  inp1=P  carry=CIN  dbl_ovrd=off  block_type=constant
      settings: block_version=VER_STRING_GOES_HERE
  Register : xbsIndex_r4/Register  init=0  rst=on  en=on  dbl_ovrd=off  block_type=register
      settings: xl_area=[0, 0, 0, 0, 0, 0, 0]  block_version=VER_STRING_GOES_HERE
  D : Outport
  wires:
  Constant2/1 -> Register/1
  R/1 -> Register/2
  S/1 -> Register/3
  Register/1 -> D/1

== system ofdm_txrx_alamouti/OFDM Rx Alamouti/Equalizer & Packetizer/Pilot_Extractor/posedge ==
  D : Inport  Orientation=down
  Inverter : xbsIndex_r4/Inverter  en=off  latency=1  dbl_ovrd=off  block_type=inv
      settings: block_version=VER_STRING_GOES_HERE
  Logical1 : xbsIndex_r4/Logical  logical_function=AND  inputs=2  en=off  latency=0  precision=Full  arith_type=Unsigned  n_bits=8  bin_pt=2  align_bp=on  dbl_ovrd=off  block_type=logical
      settings: xl_area=[1 0 0 1 0 0 0]  block_version=VER_STRING_GOES_HERE
  Q : Outport
  wires:
  Inverter/1 -> Logical1/2
  D/1 -> Logical1/1, Inverter/1
  Logical1/1 -> Q/1

== system ofdm_txrx_alamouti/OFDM Rx Alamouti/FFT & Chan Est/Addr Gen/Oscillator1 ==
  en : Inport
  rst : Inport  Port=2
  Assert : xbsIndex_r4/Assert  Orientation=left  assert_type=on  type_source=Explicitly  arith_type=Boolean  n_bits=16  bin_pt=14  assert_rate=on  rate_source=Explicitly  period=2  output_port=on  block_type=assert
      settings: infoedit=Asserts a user-defined sample rate and/or type on a signal.<P><P>Hardware notes: In hardware this block costs nothing.  block_version=VER_STRING_GOES_HERE
  Inverter1 : xbsIndex_r4/Inverter  Orientation=left  en=off  latency=0  dbl_ovrd=off  block_type=inv
      settings: block_version=VER_STRING_GOES_HERE
  Register : xbsIndex_r4/Register  init=0  rst=on  en=on  dbl_ovrd=off  block_type=register
      settings: block_version=VER_STRING_GOES_HERE
  Q : Outport
  wires:
  Register/1 -> Assert/1, Q/1
  en/1 -> Register/3
  Inverter1/1 -> Register/1
  Assert/1 -> Inverter1/1
  rst/1 -> Register/2

== system ofdm_txrx_alamouti/OFDM Rx Alamouti/FFT & Chan Est/Addr Gen/Posedge Det ==
  D : Inport
  Delay : xbsIndex_r4/Delay  en=off  latency=1  dbl_ovrd=off  reg_retiming=off  block_type=delay
      settings: infoedit=Hardware notes: A delay line is a chain, each link of which is an SRL16 followed by a flip-flop. If register retiming is enabled, the delay line is a chain of flip-flops.  xl_area=[1 1 0 0 0 0 0]  block_version=VER_STRING_GOES_HERE
  Inverter : xbsIndex_r4/Inverter  en=off  latency=0  dbl_ovrd=off  block_type=inv
      settings: xl_area=[0 0 0 0 0 0 0]  block_version=VER_STRING_GOES_HERE
  Logical : xbsIndex_r4/Logical  logical_function=AND  inputs=2  en=off  latency=0  precision=Full  arith_type=Unsigned  n_bits=8  bin_pt=2  align_bp=on  dbl_ovrd=off  block_type=logical
      settings: xl_area=[1 0 0 1 0 0 0]  block_version=VER_STRING_GOES_HERE
  Q : Outport
  wires:
  Delay/1 -> Inverter/1
  Inverter/1 -> Logical/2
  D/1 -> Logical/1, Delay/1
  Logical/1 -> Q/1

== system ofdm_txrx_alamouti/OFDM Rx Alamouti/FFT & Chan Est/Addr Gen/S-R Latch ==
  S : Inport
  R : Inport  Port=2
  Constant2 : xbsIndex_r4/Constant  arith_type=Boolean  const=1  n_bits=1  bin_pt=0  explicit_period=off  period=1  equ=P=C  opselect=C  inp2=PCIN>>17  opr=+  inp1=P  carry=CIN  dbl_ovrd=off  block_type=constant
      settings: block_version=VER_STRING_GOES_HERE
  Register : xbsIndex_r4/Register  init=0  rst=on  en=on  dbl_ovrd=off  block_type=register
      settings: xl_area=[0, 0, 0, 0, 0, 0, 0]  block_version=VER_STRING_GOES_HERE
  D : Outport
  wires:
  Constant2/1 -> Register/1
  R/1 -> Register/2
  S/1 -> Register/3
  Register/1 -> D/1

== system ofdm_txrx_alamouti/OFDM Rx Alamouti/FFT & Chan Est/Addr_Generator/FFT Start Gen ==
  WriteAddr : Inport
  Wr Slot : Inport  Port=2
  AddSub1 : xbsIndex_r4/AddSub  mode=Addition  use_carryin=off  use_carryout=off  en=off  latency=0  precision=User Defined  arith_type=Unsigned  n_bits=ceil(log2(max_num_subcarriers))+1  bin_pt=0  quantization=Truncate  overflow=Wrap  dbl_ovrd=off  use_behavioral_HDL=on  pipelined=off  use_rpm=on  block_type=addsub
      settings: xl_area=[4 0 0 6 0 0 0]  block_version=VER_STRING_GOES_HERE
  Delay : xbsIndex_r4/Delay  en=off  latency=2  dbl_ovrd=off  reg_retiming=off  block_type=delay
      settings: infoedit=Hardware notes: A delay line is a chain, each link of which is an SRL16 followed by a flip-flop. If register retiming is enabled, the delay line is a chain of flip-flops.  block_version=VER_STRING_GOES_HERE
  Display{,1..3} (4 alike) : Display
  Logical1 : xbsIndex_r4/Logical  logical_function=AND  inputs=2  en=off  latency=0  precision=Full  arith_type=Unsigned  n_bits=16  bin_pt=0  align_bp=on  dbl_ovrd=off  block_type=logical
      settings: block_version=VER_STRING_GOES_HERE
  Logical2 : xbsIndex_r4/Logical  logical_function=OR  inputs=2  en=off  latency=0  precision=Full  arith_type=Unsigned  n_bits=16  bin_pt=0  align_bp=on  dbl_ovrd=off  block_type=logical
      settings: block_version=VER_STRING_GOES_HERE
  Relational1 : xbsIndex_r4/Relational  mode=a=b  en=off  latency=0  dbl_ovrd=off  block_type=relational
      settings: block_version=VER_STRING_GOES_HERE
  Shift : xbsIndex_r4/Shift  shift_dir=Right  shift_bits=1  en=off  latency=0  precision=Full  arith_type="Signed  (2's comp)"  n_bits=16  bin_pt=14  quantization=Truncate  overflow=Wrap  dbl_ovrd=off  block_type=shift
      settings: block_version=VER_STRING_GOES_HERE
  Up Sample1 : xbsIndex_r4/Up Sample  sample_ratio=2  copy_samples=off  dbl_ovrd=off  block_type=usamp
      settings: infoedit="Up samples input data.  Inserted values  can be zeros or copies of the most recent input sample.<P><P>Hardware notes: No hardware is needed if inserted values are copies of the input sample; otherwise, a mux and single bit flip-flop are used."  block_version=VER_STRING_GOES_HERE
  done{2,7..9} (4 alike) : xbsIndex_r4/Gateway Out  hdl_port=off  timing_constraint=None  locs_specified=off  LOCs={}  block_type=gatewayout
      settings: infoedit="Gateway out block.  Converts Xilinx fixed point inputs into ouputs of type Simulink integer, double, or fixed point.<P><P>Hardware notes:  In hardware these blocks become top level output ports or are discarded, depending on how they are configured."  xl_area=[0 0 0 0 1 0 0]  block_version=VER_STRING_GOES_HERE
  Start : Outport
  wires:
  [regRX_numSubcarriers] -> Shift/1, done8/1
  Logical1/1 -> Logical2/1, Delay/1
  Up Sample1/1 -> Relational1/1
  Delay/1 -> Logical2/2
  Shift/1 -> AddSub1/1, done7/1
  [regRx_symbolTimingOffset] -> AddSub1/2, done9/1
  done2/1 -> Display/1
  done7/1 -> Display1/1
  done8/1 -> Display2/1
  done9/1 -> Display3/1
  AddSub1/1 -> done2/1, Relational1/2
  WriteAddr/1 -> Up Sample1/1
  Logical2/1 -> Start/1
  Relational1/1 -> Logical1/2
  Wr Slot/1 -> Logical1/1

== system ofdm_txrx_alamouti/OFDM Rx Alamouti/FFT & Chan Est/Addr_Generator/Oscillator ==
  en : Inport
  rst : Inport  Port=2
  Assert : xbsIndex_r4/Assert  Orientation=left  assert_type=on  type_source=Explicitly  arith_type=Boolean  n_bits=16  bin_pt=14  assert_rate=on  rate_source=Explicitly  period=4  output_port=on  block_type=assert
      settings: infoedit=Asserts a user-defined sample rate and/or type on a signal.<P><P>Hardware notes: In hardware this block costs nothing.  block_version=VER_STRING_GOES_HERE
  Inverter1 : xbsIndex_r4/Inverter  Orientation=left  en=off  latency=0  dbl_ovrd=off  block_type=inv
      settings: block_version=VER_STRING_GOES_HERE
  Register1 : xbsIndex_r4/Register  init=0  rst=on  en=on  dbl_ovrd=off  block_type=register
      settings: block_version=VER_STRING_GOES_HERE
  Q : Outport
  wires:
  rst/1 -> Register1/2
  Assert/1 -> Inverter1/1
  Inverter1/1 -> Register1/1
  en/1 -> Register1/3
  Register1/1 -> Q/1, Assert/1

== system ofdm_txrx_alamouti/OFDM Rx Alamouti/FFT & Chan Est/Addr_Generator/Posedge ==
  D : Inport
  Delay : xbsIndex_r4/Delay  en=off  latency=1  dbl_ovrd=off  reg_retiming=off  block_type=delay
      settings: infoedit=Hardware notes: A delay line is a chain, each link of which is an SRL16 followed by a flip-flop. If register retiming is enabled, the delay line is a chain of flip-flops.  xl_area=[1 1 0 0 0 0 0]  block_version=VER_STRING_GOES_HERE
  Inverter : xbsIndex_r4/Inverter  en=off  latency=0  dbl_ovrd=off  block_type=inv
      settings: xl_area=[0 0 0 0 0 0 0]  block_version=VER_STRING_GOES_HERE
  Logical : xbsIndex_r4/Logical  logical_function=AND  inputs=2  en=off  latency=0  precision=Full  arith_type=Unsigned  n_bits=8  bin_pt=2  align_bp=on  dbl_ovrd=off  block_type=logical
      settings: xl_area=[1 0 0 1 0 0 0]  block_version=VER_STRING_GOES_HERE
  Q : Outport
  wires:
  Delay/1 -> Inverter/1
  Inverter/1 -> Logical/2
  D/1 -> Logical/1, Delay/1
  Logical/1 -> Q/1

== system ofdm_txrx_alamouti/OFDM Rx Alamouti/FFT & Chan Est/Channel Estimation/Bus Creator ==
  BA-I : Inport
  BA-Q : Inport  Port=2
  AA-I : Inport  Port=3
  AA-Q : Inport  Port=4
  Bus Creator1 : BusCreator  Inputs=2
  Bus Creator2 : BusCreator  Inputs=2
  I : Outport
  Q : Outport  Port=2
  wires:
  Bus Creator1/1 -> I/1
  Bus Creator2/1 -> Q/1
  AA-Q/1 -> Bus Creator2/1
  BA-Q/1 -> Bus Creator2/2
  AA-I/1 -> Bus Creator1/1
  BA-I/1 -> Bus Creator1/2

== system ofdm_txrx_alamouti/OFDM Rx Alamouti/FFT & Chan Est/Channel Estimation/H_A-A Channel ==
  I : Inport
  Q : Inport  Port=2
  Est_WE : Inport  Port=3
  Est_Reset : Inport  Port=4
  Xk_Index : Inport  Port=5
  I Estimate Buffer : Subsystem
  Q Estimate Buffer : Subsystem
  EstI : Outport
  EstQ : Outport  Port=2
  wires:
  I/1 -> I Estimate Buffer/1(Est_Data)
  Xk_Index/1 -> I Estimate Buffer/2(Est_Addr), Q Estimate Buffer/2(Est_Addr)
  I Estimate Buffer/1(h[n]) -> EstI/1
  Est_WE/1 -> I Estimate Buffer/3(Est_WE), Q Estimate Buffer/3(Est_WE)
  Est_Reset/1 -> I Estimate Buffer/4(Rst), Q Estimate Buffer/4(Rst)
  Q Estimate Buffer/1(h[n]) -> EstQ/1
  Q/1 -> Q Estimate Buffer/1(Est_Data)

== system ofdm_txrx_alamouti/OFDM Rx Alamouti/FFT & Chan Est/Channel Estimation/H_B-A Channel ==
  I : Inport
  Q : Inport  Port=2
  Est_WE : Inport  Port=3
  Est_Reset : Inport  Port=4
  Xk_Index : Inport  Port=5
  I Estimate Buffer : Subsystem
  Q Estimate Buffer : Subsystem
  EstI : Outport
  EstQ : Outport  Port=2
  wires:
  I/1 -> I Estimate Buffer/1(Est_Data)
  Xk_Index/1 -> I Estimate Buffer/2(Est_Addr), Q Estimate Buffer/2(Est_Addr)
  I Estimate Buffer/1(h[n]) -> EstI/1
  Est_WE/1 -> I Estimate Buffer/3(Est_WE), Q Estimate Buffer/3(Est_WE)
  Est_Reset/1 -> I Estimate Buffer/4(Rst), Q Estimate Buffer/4(Rst)
  Q Estimate Buffer/1(h[n]) -> EstQ/1
  Q/1 -> Q Estimate Buffer/1(Est_Data)
